simulink model "nonlinear_double_track_model"  (R2024b)
settings: Solver=VariableStepAuto  StartTime=0.0  StopTime=5  FixedStep=0.01  RelTol=1e-4
undefined symbols (not defined in the file or in its folder): a, b, balance_bar_ratio_front, brake_pedal_motion_ratio, cg_height, disc_radius_front, disc_radius_rear, front_mass_distribution, g, gear_ratio, Jeq, Ki_yaw, Kp_yaw, mass_total, max_pedal_force, mc_diameter_front, mc_diameter_rear, num_pistons_front, num_pistons_rear, pad_friction_front, pad_friction_rear, pad_height_front, pad_height_rear, piston_radius_front, piston_radius_rear, static_toe_front, static_toe_rear, tire_loaded_radius, tire_mu, tire_mu_correction_factor, tlltd, toe_deflection_rear, track_width_front, track_width_rear, wheelbase, yaw_polar_inertia
libraries: simulink_extras x12, checkpacklib x1, slpidlib x1
(part 5 of 6)

== system nonlinear_double_track_model/Vehicle Plant Model/D2704 Tire Model/Goodyear D2704 Tire Model3 ==
  SL (unitless) : Inport
  SA (deg) : Inport  Port=2
  Fz (N) : Inport  Port=3
  "Fx vs SL, SA, Fz, IA = 2 deg, P = 8 psi" : Lookup_n-D  NumberOfTableDimensions=3  BreakpointsForDimension1=[-0.3 -0.28 -0.26 -0.24 -0.22 -0.2 -0.18 -0.16 -0.14 -0.12 -0.1 -0.08 -0.06 -0.04 -0.02 0 0.02 0.04 0.06 0.08 0.1 0.12 0.14 0.16 0.18 0.2 0.22 0.24 0.26 0.28 0.3]  BreakpointsForDimension2=[-13 -12.75 -12.5 -12.25 -12 -11.75 -11.5 -11.25 -11 -10.75 -10.5 -10.25 -10 -9.75 -9.5 -9.25 -9 -8.75 -8.5 -8.25 -8 -7.75 -7.5 -7.25 -7 -6.75 -6.5 -6.25 -6 -5.75 -5.5 -5.25 -5 -4.75 -4.5 -4.25 -4 -3.75 -3.5 -3.25 -3 -2.75 -2.5 -2.25 -2 -1.75 -1.5 -1.25 -1 -0.75 -0.5 -0.25 0 0.25 0.5 0.75 1 1.25 1.5 1.75 2 2.25 2.5 2.75 3 3.25 3.5 3.75 4 4.25 4.5 4.75 5 5.25 5.5 5.75 6 6.25 6.5 6.75 7 7.25 7.5 7.75 8 8.25 8.5 8.75 9 9.25 9.5 9.75 10 10.25 10.5 10.75 11 11.25 11.5 11.75 12 12.25 12.5 12.75 13]  BreakpointsForDimension3=[-1600 -1575 -1550 -1525 -1500 -1475 -1450 -1425 -1400 -1375 -1350 -1325 -1300 -1275 -1250 -1225 -1200 -1175 -1150 -1125 -1100 -1075 -1050 -1025 -1000 -975 -950 -925 -900 -875 -850 -825 -800 -775 -750 -725 -700 -675 -650 -625 -600 -575 -550 -525 -500 -475 -450 -425 -400 -375 -350 -325 -300 -275 -250 -225 -200 -175 -150 -125 -100 -75 -50 -25 0]  Table=reshape([-2094.90302308082 -2044.60510800287 -1985.73071384121 -1918.27439725877 -1842.1402862582 -1756.84656286299 -1661.29061799257 -1553.63258235516 -1431.33334002954 -1291.38149059854 -1130.74000248012 -947.004842614647 -739.169925181963 -508.223707777651 -256.977221234241 0 297.915318056302 584.588137680178 859.994427735328 1116.49350065739 1349.53359264341 1557.22689451721 1739.92089057765 1899.55005155979 2038.87698776631 2160.81021724911 2267.94271905856 2362.35170421901 2445.6081877889 2518.90595421019 2583.2254130159 -2116.43071148897 -2067.13353380789 -2009.08009328621 -1942.34483537959 -1866.91575084034 -1782.36192611476 -1687.56974122987 -1580.60752846602 -1458.75340921278 -1318.72432472422 -1157.14739547866 -971.277777657572 -759.858817427872 -523.823735295896 -266.167642712169 0 303.855713102641 597.94860064122 879.762422103886 1141.25631035796 1377.73066571245 1587.36764461283 1770.75531143211 1930.16293104293 2068.69430297003 2189.53559915636 2295.4614648088 2388.63895047053 2470.66277264765 2542.71547220426 2605.7579328179 -2138.25995695721 -2089.99615012123 -2032.78252001018 -1966.78460083662 -1892.08727381387 -1808.32093220632 -1714.36646726816 -1608.19984080208 -1486.90772807908 -1346.9156260666 -1184.48179081211 -996.476188316672 -781.344174105178 -539.925100086236 -275.375009106421 0 310.567028317014 612.262145718792 900.646564701561 1167.22264930368 1407.12360912311 1618.61043754914 1802.53964964662 1961.55108159679 2099.12138529522 2218.73370553496 2323.34985446452 2415.22220881249 2495.95998434884 2566.724887499 2628.45101082459 -2160.39858569596 -2113.2022030637 -2056.84639012436 -1991.60027895332 -1917.66015235994 -1834.72916517197 -1741.6889882629 -1636.42337722606 -1515.81947405891 -1375.99134484314 -1212.79345334728 -1022.66205411425 -803.691845476831 -556.58844984992 -284.666335033056 0 318.036855838273 627.56450776448 922.698399885035 1194.44723766795 1437.76124013535 1650.99146442379 1835.29434882415 1993.72021923252 2130.15331547848 2248.39404697328 2351.59602520288 2442.09075730179 2521.49112778985 2590.92722582293 2651.29845058211 -2182.85546192761 -2136.76229866856 -2081.28143088532 -2016.79958978189 -1943.64060436953 -1861.59290703014 -1769.54595259005 -1665.29230171156 -1545.51232451255 -1405.98877743731 -1242.13569235429 -1049.90286487706 -826.976193927666 -573.887227422349 -294.127213308017 0 326.238413786205 643.885560432785 945.968461073959 1222.98548366616 1469.69320981638 1684.54699055717 1869.03883469087 2026.67419833273 2161.78306671345 2278.50434606455 2380.18693231547 2469.23330733235 2547.24740642872 2615.31566200897 2674.29423746624 -2205.64063611533 -2160.68863752915 -2106.09896810827 -2042.3916731244 -1970.0360717278 -1888.91942986099 -1797.94668588952 -1694.82117726415 -1576.01040089944 -1436.94640394917 -1272.56470027782 -1078.27155512912 -851.279903158488 -591.905557100224 -303.849037997001 0 335.143593595636 661.251796097133 970.506713617727 1252.89358562114 1502.97001838737 1719.31328649171 1903.79135661581 2060.4148192612 2194.00136821211 2309.05051139027 2409.10842392583 2496.63813508685 2573.22006879697 2639.88365092307 2697.4326309563 -2228.76551210975 -2184.99529024385 -2131.31224643017 -2068.38743928916 -1996.85560495935 -1916.7173766347 -1826.90149209347 -1725.02511300764 -1607.33822580759 -1468.90368726774 -1304.13930489404 -1107.84631789694 -876.693633353155 -610.736167494972 -313.922557903896 0 344.729666854667 679.6887315254 996.363076216134 1284.22865921428 1537.64303715504 1755.32655774919 1939.56882261523 2094.94163396947 2226.7965846862 2340.01664730156 2438.34535985275 2524.29325276286 2599.40058503619 2664.6250780504 2720.70826583422 -2252.24303533435 -2209.69852186673 -2156.93681430322 -2094.80000184354 -2024.11035046356 -1944.99725445979 -1856.42205751959 -1755.91998564674 -1639.52070708227 -1501.90083783596 -1336.92062869901 -1138.71029748288 -903.315603693779 -630.478808009042 -324.434879252478 0 354.982519689571 699.222756382071 1023.58790575648 ...(+3574022 chars)])  InterpMethod=Cubic spline  InternalRulePriority=Speed  RndMeth=Simplest
      settings: InputPortMap=u0,u1,u2
  "Fy vs SL, SA, Fz, IA = 2 deg, P = 8 psi" : Lookup_n-D  NumberOfTableDimensions=3  BreakpointsForDimension1=[-0.3 -0.28 -0.26 -0.24 -0.22 -0.2 -0.18 -0.16 -0.14 -0.12 -0.1 -0.08 -0.06 -0.04 -0.02 0 0.02 0.04 0.06 0.08 0.1 0.12 0.14 0.16 0.18 0.2 0.22 0.24 0.26 0.28 0.3]  BreakpointsForDimension2=[-13 -12.75 -12.5 -12.25 -12 -11.75 -11.5 -11.25 -11 -10.75 -10.5 -10.25 -10 -9.75 -9.5 -9.25 -9 -8.75 -8.5 -8.25 -8 -7.75 -7.5 -7.25 -7 -6.75 -6.5 -6.25 -6 -5.75 -5.5 -5.25 -5 -4.75 -4.5 -4.25 -4 -3.75 -3.5 -3.25 -3 -2.75 -2.5 -2.25 -2 -1.75 -1.5 -1.25 -1 -0.75 -0.5 -0.25 0 0.25 0.5 0.75 1 1.25 1.5 1.75 2 2.25 2.5 2.75 3 3.25 3.5 3.75 4 4.25 4.5 4.75 5 5.25 5.5 5.75 6 6.25 6.5 6.75 7 7.25 7.5 7.75 8 8.25 8.5 8.75 9 9.25 9.5 9.75 10 10.25 10.5 10.75 11 11.25 11.5 11.75 12 12.25 12.5 12.75 13]  BreakpointsForDimension3=[-1600 -1575 -1550 -1525 -1500 -1475 -1450 -1425 -1400 -1375 -1350 -1325 -1300 -1275 -1250 -1225 -1200 -1175 -1150 -1125 -1100 -1075 -1050 -1025 -1000 -975 -950 -925 -900 -875 -850 -825 -800 -775 -750 -725 -700 -675 -650 -625 -600 -575 -550 -525 -500 -475 -450 -425 -400 -375 -350 -325 -300 -275 -250 -225 -200 -175 -150 -125 -100 -75 -50 -25 0]  Table=reshape([1840.02487064204 1928.23739067748 2022.9996119429 2124.20496238938 2231.42168880577 2343.85501653743 2460.32164581596 2579.19844163219 2698.33060565409 2814.92769562247 2925.50435171862 3025.90691426595 3111.36599154229 3176.19362374231 3211.46137476601 3193.50065964949 3093.9924405899 2948.15771683353 2785.64952211612 2619.74432768095 2458.45293535555 2306.82384272125 2167.55675705262 2041.48205091641 1928.13313362129 1826.32067762761 1734.58468176615 1651.47600023669 1575.69515586203 1506.14364152493 1441.93103899025 1814.72170923149 1903.09544570806 1998.18142378198 2099.90300616636 2207.84394070074 2321.21456991475 2438.83372584397 2559.07745701476 2679.77461400567 2798.08217328007 2910.40865685295 3012.44000818923 3099.21399571101 3164.83290741187 3200.00564389336 3180.27044322814 3077.31871737184 2926.88808438963 2759.85617303807 2590.12934805822 2426.00347966791 2272.61639550241 2132.59288993521 2006.57061651039 1893.84162802951 1792.98966221976 1702.37691039335 1620.43122573368 1545.7750262052 1477.26412119692 1413.98537889148 1789.02584933522 1877.51099168842 1972.86949277714 2075.05580912052 2183.66779928684 2297.91845986601 2416.62778304052 2538.17231526947 2660.36578784949 2780.30877682504 2894.2885089739 2997.79724703108 3085.62058006701 3151.5469590728 3185.7320619788 3163.27115174717 3057.52395156472 2903.13292162416 2731.88492650101 2558.52363098889 2391.73162837159 2236.77649242721 2096.21199054649 1970.46709600361 1858.57210512611 1758.86938774582 1669.53773926544 1588.88279155836 1515.45237988148 1448.06066634668 1385.77566735203 1762.94030261445 1851.48592426849 1947.06467140476 2049.66343236169 2158.89233316797 2273.96386747562 2393.69801758355 2516.47377267999 2640.09215796997 2761.59462450584 2877.13221560315 2981.96929360827 3070.57967333693 3136.34911468709 3168.80274477678 3143.0398537961 3034.75065852147 2876.87080645805 2701.68714918111 2524.87564091575 2355.59098856597 2199.26869611299 2058.39289912018 1933.16435583375 1822.32832271548 1723.97037015694 1636.08092838263 1556.84538193488 1484.74148821195 1418.54637789806 1357.31340014981 1736.46904982997 1825.02317028426 1920.76898273086 2023.72739556707 2133.51840588187 2249.34998333326 2370.04068617828 2493.97467574741 2618.94418576386 2741.93036870748 2858.93412996249 2964.95780875661 3054.10588305411 3119.29482993873 3149.4558045869 3120.09372367422 3009.21617207134 2848.12054173067 2669.23293562982 2489.14343818322 2317.54080481295 2160.0619011062 2019.11873941286 1894.65972663733 1785.11833849102 1688.30685343062 1602.02321457661 1524.33595073127 1453.6583183823 1388.73560233353 1328.61095729156 1709.61709762082 1798.12674033636 1893.98568127416 1997.25078311091 2107.54883868369 2224.07819226553 2345.65425663592 2470.67004729976 2596.91476639964 2721.31012208979 2839.69450898201 2946.7750936539 3036.23256974636 3100.46912397067 3127.93309102459 3094.81223072599 2981.14036585371 2816.92861321491 2634.50927653284 2451.29450135505 2277.54607528648 2119.12959577811 1978.37730928444 1854.95544435369 1746.95488959539 1651.89706111063 1567.38444089778 1491.37377145182 1422.22054263375 1358.64392023951 1299.6815783041 1682.3905423428 1770.80178616049 1866.71931715969 1970.23836138874 2080.98859512618 2198.15228224396 2320.53957886578 2446.55717274852 2573.99920455749 2699.73130675602 2819.41920649867 2927.4434179468 3017.00939042203 3079.97491026689 3104.44438405104 3067.47003997593 2950.70984402845 2783.35757693586 2597.51776188914 2411.30530851403 2235.57756925436 2076.45010539392 1936.1614966062 1814.05912884674 1707.85579546264 1614.76344902637 1532.18767386736 1457.98047293431 1390.44753612536 1328.2881264194 1270.53933041891 1654.79664345893 1743.05466524956 1838.97580538465 1942.69671100155 2053.84499336356 2171.57868392439 2294.70007882521 2421.63569394538 2550.19517187492 2677.19443746102 2798.11922137604 2906.99412101247 2996.49980901749 3057.92470229724 3079.15611635802 3038.22793111669 2918.0666847771 2747.47787775665 2558.27233711907 ...(+3682675 chars)])  InterpMethod=Cubic spline  InternalRulePriority=Speed  RndMeth=Simplest
      settings: InputPortMap=u0,u1,u2
  "Mz vs SL, SA, Fz, IA = 2 deg, P = 8 psi" : Lookup_n-D  NumberOfTableDimensions=3  BreakpointsForDimension1=[-0.3 -0.28 -0.26 -0.24 -0.22 -0.2 -0.18 -0.16 -0.14 -0.12 -0.1 -0.08 -0.06 -0.04 -0.02 0 0.02 0.04 0.06 0.08 0.1 0.12 0.14 0.16 0.18 0.2 0.22 0.24 0.26 0.28 0.3]  BreakpointsForDimension2=[-13 -12.75 -12.5 -12.25 -12 -11.75 -11.5 -11.25 -11 -10.75 -10.5 -10.25 -10 -9.75 -9.5 -9.25 -9 -8.75 -8.5 -8.25 -8 -7.75 -7.5 -7.25 -7 -6.75 -6.5 -6.25 -6 -5.75 -5.5 -5.25 -5 -4.75 -4.5 -4.25 -4 -3.75 -3.5 -3.25 -3 -2.75 -2.5 -2.25 -2 -1.75 -1.5 -1.25 -1 -0.75 -0.5 -0.25 0 0.25 0.5 0.75 1 1.25 1.5 1.75 2 2.25 2.5 2.75 3 3.25 3.5 3.75 4 4.25 4.5 4.75 5 5.25 5.5 5.75 6 6.25 6.5 6.75 7 7.25 7.5 7.75 8 8.25 8.5 8.75 9 9.25 9.5 9.75 10 10.25 10.5 10.75 11 11.25 11.5 11.75 12 12.25 12.5 12.75 13]  BreakpointsForDimension3=[-1600 -1575 -1550 -1525 -1500 -1475 -1450 -1425 -1400 -1375 -1350 -1325 -1300 -1275 -1250 -1225 -1200 -1175 -1150 -1125 -1100 -1075 -1050 -1025 -1000 -975 -950 -925 -900 -875 -850 -825 -800 -775 -750 -725 -700 -675 -650 -625 -600 -575 -550 -525 -500 -475 -450 -425 -400 -375 -350 -325 -300 -275 -250 -225 -200 -175 -150 -125 -100 -75 -50 -25 0]  Table=reshape([90.2754120059503 88.1429292073834 84.9697193890524 80.6919096498076 75.2765817089414 68.7073649512466 60.9702465853157 52.0508366743801 41.951356596013 30.7318857491877 18.5786825100366 5.9007602577717 -6.55337826918336 -17.6016485641335 -25.7397693547386 -30.3591212781865 -32.5605654761185 -31.0215499578796 -26.1550524233271 -19.3159722685142 -11.5934362273791 -3.69815660314923 3.95443106623218 11.1441438225302 17.7673094158714 23.7833573274733 29.1825973451514 33.9705650758661 38.1642269644111 41.7941663503087 44.9064921157562 91.0071974549012 88.9430874601252 85.8067944884063 81.5327045857263 76.0889110134167 69.4594356571222 61.6260475703563 52.5626799398824 42.2515209140693 30.7254332909954 18.1400916006396 4.87904162592468 -8.31299977180149 -20.2083918505678 -29.1646189515015 -34.2375209035443 -36.1365332464212 -33.9169409170093 -28.3162969806971 -20.8213212402969 -12.5655684564718 -4.2595395291746 3.70011612339612 11.1160488775265 17.9049128250043 24.0416127970946 29.5267309939375 34.3717412760986 38.5970047586746 42.2360389665441 45.3388191795892 91.7540125610532 89.7646374162113 86.6704618641921 82.4045518332793 76.9371165169358 70.2538170709727 62.3329353934346 53.1364957731537 42.6251980210659 30.801687679157 17.7859370232071 3.92808029498792 -10.0421202895226 -22.8661771972555 -32.7807759284118 -38.4339274791189 -39.908344862823 -36.8713601157211 -30.4565839549059 -22.2647963041792 -13.4594810637262 -4.74039800438405 3.52168027825161 11.1565177497793 18.1034483513677 24.3539653900702 29.9191931186837 34.8162475014215 39.0683535354632 42.7115717362286 45.7997133204047 92.5151366783918 90.6071241703061 87.5603373931508 83.3069948423436 77.8207284942383 71.0903447554368 63.0915874304408 53.7744998873592 43.0769764681236 30.9685727140455 17.5284879455803 3.06534656355649 -11.7173798347212 -25.5434503079543 -36.5346131492758 -42.8518002664191 -43.8225390378054 -39.8534909549646 -32.5527043307916 -23.6288194210149 -14.2624083647699 -5.13187244597006 3.42505051518231 11.2694007496336 18.3653739934995 24.7220093029982 30.3610961482541 35.304937687347 39.5789360868195 43.2212050383545 46.2893533355466 93.2897569558088 91.4700190137809 88.4759396609219 84.2394112159651 78.7390248483717 71.9685310843933 63.9023229727705 54.4785653295514 43.6111990256313 31.2340140780942 17.380532442086 2.30978065611704 -13.312278957695 -28.2021637405824 -40.3599785162636 -47.3864013949711 -47.8131257784886 -42.8255093599312 -34.5782734686439 -24.8942739647322 -14.9609602382742 -5.42496378130933 3.41606572064388 11.4583688430397 18.6929451105559 25.1471502898125 30.853401317379 35.8385592804597 40.1293410852017 43.7653140455817 46.8078453875779 94.0769632674007 92.3527212570868 89.4166920158667 85.2010057825199 79.6910105186095 72.887530395225 64.7650516901327 55.2501469592856 44.2318525371962 31.6057850850698 17.3551748728868 1.68152089140104 -14.79771183493 -30.7996776752694 -44.1871944451062 -51.9460718284387 -51.8105818522019 -45.7449765819806 -36.5042522986142 -26.0407610179491 -15.5413067063706 -5.61066932100853 3.50038278111926 11.7268506203233 19.088171394045 25.6305757690055 31.3968992062484 36.4177427971155 40.7200779993921 44.3442037849248 47.3552157028956 94.8757423649718 93.2545613233683 90.3819271402906 86.1908057719237 80.6753981926589 73.8461050965166 65.6792221374364 56.0902029080121 44.9424479338842 32.0913303128456 17.4655929304367 1.20156818694059 -16.1426019238385 -33.2907291064753 -47.9478798201678 -56.4520338036573 -55.7466674740533 -48.5667981419596 -38.2995681026903 -27.0469160091396 -15.9893996214415 -5.68013815962139 3.68337430895425 12.0779664193314 19.5527730317335 26.1732245370504 31.9921900400474 37.0429916232919 41.3515724524907 44.9581039809738 47.9314022790409 95.6849708577577 94.1748051873523 91.3708946426158 87.2076586661947 81.6905910150937 74.8425938015394 66.6437717208754 56.9991146045998 45.7458919635848 32.697568309588 17.7247559039728 0.891398306569926 -17.3145692635252 -35.6291721027867 ...(+3621783 chars)])  InterpMethod=Cubic spline  InternalRulePriority=Speed  RndMeth=Simplest
      settings: InputPortMap=u0,u1,u2
  Relaxation Length : TransferFcn  Denominator=[0.02 1]
  Relaxation Length2 : TransferFcn  Denominator=[0.02 1]
  Fy : Outport
  Fx : Outport  Port=2
  Mz : Outport  Port=3
  wires:
  Fz (N)/1 -> "Fx vs SL, SA, Fz, IA = 2 deg, P = 8 psi"/3, "Mz vs SL, SA, Fz, IA = 2 deg, P = 8 psi"/3, "Fy vs SL, SA, Fz, IA = 2 deg, P = 8 psi"/3
  SL (unitless)/1 -> "Fx vs SL, SA, Fz, IA = 2 deg, P = 8 psi"/1, "Mz vs SL, SA, Fz, IA = 2 deg, P = 8 psi"/1, "Fy vs SL, SA, Fz, IA = 2 deg, P = 8 psi"/1
  SA (deg)/1 -> "Fx vs SL, SA, Fz, IA = 2 deg, P = 8 psi"/2, "Mz vs SL, SA, Fz, IA = 2 deg, P = 8 psi"/2, "Fy vs SL, SA, Fz, IA = 2 deg, P = 8 psi"/2
  Relaxation Length/1 -> Fy/1  "Fy"
  "Fx vs SL, SA, Fz, IA = 2 deg, P = 8 psi"/1 -> Fx/1
  Relaxation Length2/1 -> Mz/1
  "Fy vs SL, SA, Fz, IA = 2 deg, P = 8 psi"/1 -> Relaxation Length/1
  "Mz vs SL, SA, Fz, IA = 2 deg, P = 8 psi"/1 -> Relaxation Length2/1
